simulink model "SOC_2023b"  (R2023b)
settings: Solver=VariableStepAuto  StartTime=0.0  StopTime=10.0  RelTol=1e-3
undefined symbols (not defined in the file or in its folder): Initial_SOC
(part 4 of 187)

== system SOC_2023b/SOC/Multi-Temperature SOC EKF51 ==
  note: McMaster Automotive Research Centre - MARC
      | Electrical Engineer: Romulo Navega Vieira, M.Sc and MBA - PhD Candidate
  Current : Inport
  Voltage : Inport  Port=2
  Temperature : Inport  Port=3
  Data Store Write1 : DataStoreWrite  DataStoreName=HVBat_SOCcell051  IndexOptions=Index vector (dialog)  Indices=1  OutputSizes=1
  Extended Kalman Filter - Multi Temperature EKF : Subsystem
  Gain : Gain  Gain=100
  Saturation : Saturate  UpperLimit=100  LowerLimit=0
  HVBat_SOCcell051 : Outport
  wires:
  Voltage/1 -> Extended Kalman Filter - Multi Temperature EKF/2(Voltage)
  Current/1 -> Extended Kalman Filter - Multi Temperature EKF/1(Current)
  Temperature/1 -> Extended Kalman Filter - Multi Temperature EKF/3(Temperature)
  Extended Kalman Filter - Multi Temperature EKF/1(SOC) -> Gain/1
  Gain/1 -> Saturation/1
  Saturation/1 -> HVBat_SOCcell051/1, Data Store Write1/1

== system SOC_2023b/SOC/Multi-Temperature SOC EKF52 ==
  note: McMaster Automotive Research Centre - MARC
      | Electrical Engineer: Romulo Navega Vieira, M.Sc and MBA - PhD Candidate
  Current : Inport
  Voltage : Inport  Port=2
  Temperature : Inport  Port=3
  Data Store Write1 : DataStoreWrite  DataStoreName=HVBat_SOCcell052  IndexOptions=Index vector (dialog)  Indices=1  OutputSizes=1
  Extended Kalman Filter - Multi Temperature EKF : Subsystem
  Gain : Gain  Gain=100
  Saturation : Saturate  UpperLimit=100  LowerLimit=0
  HVBat_SOCcell052 : Outport
  wires:
  Voltage/1 -> Extended Kalman Filter - Multi Temperature EKF/2(Voltage)
  Current/1 -> Extended Kalman Filter - Multi Temperature EKF/1(Current)
  Temperature/1 -> Extended Kalman Filter - Multi Temperature EKF/3(Temperature)
  Extended Kalman Filter - Multi Temperature EKF/1(SOC) -> Gain/1
  Gain/1 -> Saturation/1
  Saturation/1 -> HVBat_SOCcell052/1, Data Store Write1/1

== system SOC_2023b/SOC/Multi-Temperature SOC EKF53 ==
  note: McMaster Automotive Research Centre - MARC
      | Electrical Engineer: Romulo Navega Vieira, M.Sc and MBA - PhD Candidate
  Current : Inport
  Voltage : Inport  Port=2
  Temperature : Inport  Port=3
  Data Store Write1 : DataStoreWrite  DataStoreName=HVBat_SOCcell053  IndexOptions=Index vector (dialog)  Indices=1  OutputSizes=1
  Extended Kalman Filter - Multi Temperature EKF : Subsystem
  Gain : Gain  Gain=100
  Saturation : Saturate  UpperLimit=100  LowerLimit=0
  HVBat_SOCcell053 : Outport
  wires:
  Voltage/1 -> Extended Kalman Filter - Multi Temperature EKF/2(Voltage)
  Current/1 -> Extended Kalman Filter - Multi Temperature EKF/1(Current)
  Temperature/1 -> Extended Kalman Filter - Multi Temperature EKF/3(Temperature)
  Extended Kalman Filter - Multi Temperature EKF/1(SOC) -> Gain/1
  Gain/1 -> Saturation/1
  Saturation/1 -> HVBat_SOCcell053/1, Data Store Write1/1

== system SOC_2023b/SOC/Multi-Temperature SOC EKF54 ==
  note: McMaster Automotive Research Centre - MARC
      | Electrical Engineer: Romulo Navega Vieira, M.Sc and MBA - PhD Candidate
  Current : Inport
  Voltage : Inport  Port=2
  Temperature : Inport  Port=3
  Data Store Write1 : DataStoreWrite  DataStoreName=HVBat_SOCcell054  IndexOptions=Index vector (dialog)  Indices=1  OutputSizes=1
  Extended Kalman Filter - Multi Temperature EKF : Subsystem
  Gain : Gain  Gain=100
  Saturation : Saturate  UpperLimit=100  LowerLimit=0
  HVBat_SOCcell054 : Outport
  wires:
  Voltage/1 -> Extended Kalman Filter - Multi Temperature EKF/2(Voltage)
  Current/1 -> Extended Kalman Filter - Multi Temperature EKF/1(Current)
  Temperature/1 -> Extended Kalman Filter - Multi Temperature EKF/3(Temperature)
  Extended Kalman Filter - Multi Temperature EKF/1(SOC) -> Gain/1
  Gain/1 -> Saturation/1
  Saturation/1 -> HVBat_SOCcell054/1, Data Store Write1/1

== system SOC_2023b/SOC/Multi-Temperature SOC EKF55 ==
  note: McMaster Automotive Research Centre - MARC
      | Electrical Engineer: Romulo Navega Vieira, M.Sc and MBA - PhD Candidate
  Current : Inport
  Voltage : Inport  Port=2
  Temperature : Inport  Port=3
  Data Store Write1 : DataStoreWrite  DataStoreName=HVBat_SOCcell055  IndexOptions=Index vector (dialog)  Indices=1  OutputSizes=1
  Extended Kalman Filter - Multi Temperature EKF : Subsystem
  Gain : Gain  Gain=100
  Saturation : Saturate  UpperLimit=100  LowerLimit=0
  HVBat_SOCcell055 : Outport
  wires:
  Voltage/1 -> Extended Kalman Filter - Multi Temperature EKF/2(Voltage)
  Current/1 -> Extended Kalman Filter - Multi Temperature EKF/1(Current)
  Temperature/1 -> Extended Kalman Filter - Multi Temperature EKF/3(Temperature)
  Extended Kalman Filter - Multi Temperature EKF/1(SOC) -> Gain/1
  Gain/1 -> Saturation/1
  Saturation/1 -> HVBat_SOCcell055/1, Data Store Write1/1

== system SOC_2023b/SOC/Multi-Temperature SOC EKF56 ==
  note: McMaster Automotive Research Centre - MARC
      | Electrical Engineer: Romulo Navega Vieira, M.Sc and MBA - PhD Candidate
  Current : Inport
  Voltage : Inport  Port=2
  Temperature : Inport  Port=3
  Data Store Write1 : DataStoreWrite  DataStoreName=HVBat_SOCcell056  IndexOptions=Index vector (dialog)  Indices=1  OutputSizes=1
  Extended Kalman Filter - Multi Temperature EKF : Subsystem
  Gain : Gain  Gain=100
  Saturation : Saturate  UpperLimit=100  LowerLimit=0
  HVBat_SOCcell056 : Outport
  wires:
  Voltage/1 -> Extended Kalman Filter - Multi Temperature EKF/2(Voltage)
  Current/1 -> Extended Kalman Filter - Multi Temperature EKF/1(Current)
  Temperature/1 -> Extended Kalman Filter - Multi Temperature EKF/3(Temperature)
  Extended Kalman Filter - Multi Temperature EKF/1(SOC) -> Gain/1
  Gain/1 -> Saturation/1
  Saturation/1 -> HVBat_SOCcell056/1, Data Store Write1/1

== system SOC_2023b/SOC/Multi-Temperature SOC EKF57 ==
  note: McMaster Automotive Research Centre - MARC
      | Electrical Engineer: Romulo Navega Vieira, M.Sc and MBA - PhD Candidate
  Current : Inport
  Voltage : Inport  Port=2
  Temperature : Inport  Port=3
  Data Store Write1 : DataStoreWrite  DataStoreName=HVBat_SOCcell057  IndexOptions=Index vector (dialog)  Indices=1  OutputSizes=1
  Extended Kalman Filter - Multi Temperature EKF : Subsystem
  Gain : Gain  Gain=100
  Saturation : Saturate  UpperLimit=100  LowerLimit=0
  HVBat_SOCcell057 : Outport
  wires:
  Voltage/1 -> Extended Kalman Filter - Multi Temperature EKF/2(Voltage)
  Current/1 -> Extended Kalman Filter - Multi Temperature EKF/1(Current)
  Temperature/1 -> Extended Kalman Filter - Multi Temperature EKF/3(Temperature)
  Extended Kalman Filter - Multi Temperature EKF/1(SOC) -> Gain/1
  Gain/1 -> Saturation/1
  Saturation/1 -> HVBat_SOCcell057/1, Data Store Write1/1

== system SOC_2023b/SOC/Multi-Temperature SOC EKF58 ==
  note: McMaster Automotive Research Centre - MARC
      | Electrical Engineer: Romulo Navega Vieira, M.Sc and MBA - PhD Candidate
  Current : Inport
  Voltage : Inport  Port=2
  Temperature : Inport  Port=3
  Data Store Write1 : DataStoreWrite  DataStoreName=HVBat_SOCcell058  IndexOptions=Index vector (dialog)  Indices=1  OutputSizes=1
  Extended Kalman Filter - Multi Temperature EKF : Subsystem
  Gain : Gain  Gain=100
  Saturation : Saturate  UpperLimit=100  LowerLimit=0
  HVBat_SOCcell058 : Outport
  wires:
  Voltage/1 -> Extended Kalman Filter - Multi Temperature EKF/2(Voltage)
  Current/1 -> Extended Kalman Filter - Multi Temperature EKF/1(Current)
  Temperature/1 -> Extended Kalman Filter - Multi Temperature EKF/3(Temperature)
  Extended Kalman Filter - Multi Temperature EKF/1(SOC) -> Gain/1
  Gain/1 -> Saturation/1
  Saturation/1 -> HVBat_SOCcell058/1, Data Store Write1/1

== system SOC_2023b/SOC/Multi-Temperature SOC EKF59 ==
  note: McMaster Automotive Research Centre - MARC
      | Electrical Engineer: Romulo Navega Vieira, M.Sc and MBA - PhD Candidate
  Current : Inport
  Voltage : Inport  Port=2
  Temperature : Inport  Port=3
  Data Store Write1 : DataStoreWrite  DataStoreName=HVBat_SOCcell059  IndexOptions=Index vector (dialog)  Indices=1  OutputSizes=1
  Extended Kalman Filter - Multi Temperature EKF : Subsystem
  Gain : Gain  Gain=100
  Saturation : Saturate  UpperLimit=100  LowerLimit=0
  HVBat_SOCcell059 : Outport
  wires:
  Voltage/1 -> Extended Kalman Filter - Multi Temperature EKF/2(Voltage)
  Current/1 -> Extended Kalman Filter - Multi Temperature EKF/1(Current)
  Temperature/1 -> Extended Kalman Filter - Multi Temperature EKF/3(Temperature)
  Extended Kalman Filter - Multi Temperature EKF/1(SOC) -> Gain/1
  Gain/1 -> Saturation/1
  Saturation/1 -> HVBat_SOCcell059/1, Data Store Write1/1

== system SOC_2023b/SOC/Multi-Temperature SOC EKF6 ==
  note: McMaster Automotive Research Centre - MARC
      | Electrical Engineer: Romulo Navega Vieira, M.Sc and MBA - PhD Candidate
  Current : Inport
  Voltage : Inport  Port=2
  Temperature : Inport  Port=3
  Data Store Write1 : DataStoreWrite  DataStoreName=HVBat_SOCcell006  IndexOptions=Index vector (dialog)  Indices=1  OutputSizes=1
  Extended Kalman Filter - Multi Temperature EKF : Subsystem
  Gain : Gain  Gain=100
  Saturation : Saturate  UpperLimit=100  LowerLimit=0
  HVBat_SOCcell006 : Outport
  wires:
  Voltage/1 -> Extended Kalman Filter - Multi Temperature EKF/2(Voltage)
  Current/1 -> Extended Kalman Filter - Multi Temperature EKF/1(Current)
  Temperature/1 -> Extended Kalman Filter - Multi Temperature EKF/3(Temperature)
  Extended Kalman Filter - Multi Temperature EKF/1(SOC) -> Gain/1
  Gain/1 -> Saturation/1
  Saturation/1 -> HVBat_SOCcell006/1, Data Store Write1/1

== system SOC_2023b/SOC/Multi-Temperature SOC EKF60 ==
  note: McMaster Automotive Research Centre - MARC
      | Electrical Engineer: Romulo Navega Vieira, M.Sc and MBA - PhD Candidate
  Current : Inport
  Voltage : Inport  Port=2
  Temperature : Inport  Port=3
  Data Store Write1 : DataStoreWrite  DataStoreName=HVBat_SOCcell060  IndexOptions=Index vector (dialog)  Indices=1  OutputSizes=1
  Extended Kalman Filter - Multi Temperature EKF : Subsystem
  Gain : Gain  Gain=100
  Saturation : Saturate  UpperLimit=100  LowerLimit=0
  HVBat_SOCcell060 : Outport
  wires:
  Voltage/1 -> Extended Kalman Filter - Multi Temperature EKF/2(Voltage)
  Current/1 -> Extended Kalman Filter - Multi Temperature EKF/1(Current)
  Temperature/1 -> Extended Kalman Filter - Multi Temperature EKF/3(Temperature)
  Extended Kalman Filter - Multi Temperature EKF/1(SOC) -> Gain/1
  Gain/1 -> Saturation/1
  Saturation/1 -> HVBat_SOCcell060/1, Data Store Write1/1

== system SOC_2023b/SOC/Multi-Temperature SOC EKF61 ==
  note: McMaster Automotive Research Centre - MARC
      | Electrical Engineer: Romulo Navega Vieira, M.Sc and MBA - PhD Candidate
  Current : Inport
  Voltage : Inport  Port=2
  Temperature : Inport  Port=3
  Data Store Write1 : DataStoreWrite  DataStoreName=HVBat_SOCcell061  IndexOptions=Index vector (dialog)  Indices=1  OutputSizes=1
  Extended Kalman Filter - Multi Temperature EKF : Subsystem
  Gain : Gain  Gain=100
  Saturation : Saturate  UpperLimit=100  LowerLimit=0
  HVBat_SOCcell061 : Outport
  wires:
  Voltage/1 -> Extended Kalman Filter - Multi Temperature EKF/2(Voltage)
  Current/1 -> Extended Kalman Filter - Multi Temperature EKF/1(Current)
  Temperature/1 -> Extended Kalman Filter - Multi Temperature EKF/3(Temperature)
  Extended Kalman Filter - Multi Temperature EKF/1(SOC) -> Gain/1
  Gain/1 -> Saturation/1
  Saturation/1 -> HVBat_SOCcell061/1, Data Store Write1/1

== system SOC_2023b/SOC/Multi-Temperature SOC EKF62 ==
  note: McMaster Automotive Research Centre - MARC
      | Electrical Engineer: Romulo Navega Vieira, M.Sc and MBA - PhD Candidate
  Current : Inport
  Voltage : Inport  Port=2
  Temperature : Inport  Port=3
  Data Store Write1 : DataStoreWrite  DataStoreName=HVBat_SOCcell062  IndexOptions=Index vector (dialog)  Indices=1  OutputSizes=1
  Extended Kalman Filter - Multi Temperature EKF : Subsystem
  Gain : Gain  Gain=100
  Saturation : Saturate  UpperLimit=100  LowerLimit=0
  HVBat_SOCcell062 : Outport
  wires:
  Voltage/1 -> Extended Kalman Filter - Multi Temperature EKF/2(Voltage)
  Current/1 -> Extended Kalman Filter - Multi Temperature EKF/1(Current)
  Temperature/1 -> Extended Kalman Filter - Multi Temperature EKF/3(Temperature)
  Extended Kalman Filter - Multi Temperature EKF/1(SOC) -> Gain/1
  Gain/1 -> Saturation/1
  Saturation/1 -> HVBat_SOCcell062/1, Data Store Write1/1

== system SOC_2023b/SOC/Multi-Temperature SOC EKF63 ==
  note: McMaster Automotive Research Centre - MARC
      | Electrical Engineer: Romulo Navega Vieira, M.Sc and MBA - PhD Candidate
  Current : Inport
  Voltage : Inport  Port=2
  Temperature : Inport  Port=3
  Data Store Write1 : DataStoreWrite  DataStoreName=HVBat_SOCcell063  IndexOptions=Index vector (dialog)  Indices=1  OutputSizes=1
  Extended Kalman Filter - Multi Temperature EKF : Subsystem
  Gain : Gain  Gain=100
  Saturation : Saturate  UpperLimit=100  LowerLimit=0
  HVBat_SOCcell063 : Outport
  wires:
  Voltage/1 -> Extended Kalman Filter - Multi Temperature EKF/2(Voltage)
  Current/1 -> Extended Kalman Filter - Multi Temperature EKF/1(Current)
  Temperature/1 -> Extended Kalman Filter - Multi Temperature EKF/3(Temperature)
  Extended Kalman Filter - Multi Temperature EKF/1(SOC) -> Gain/1
  Gain/1 -> Saturation/1
  Saturation/1 -> HVBat_SOCcell063/1, Data Store Write1/1

== system SOC_2023b/SOC/Multi-Temperature SOC EKF64 ==
  note: McMaster Automotive Research Centre - MARC
      | Electrical Engineer: Romulo Navega Vieira, M.Sc and MBA - PhD Candidate
  Current : Inport
  Voltage : Inport  Port=2
  Temperature : Inport  Port=3
  Data Store Write1 : DataStoreWrite  DataStoreName=HVBat_SOCcell064  IndexOptions=Index vector (dialog)  Indices=1  OutputSizes=1
  Extended Kalman Filter - Multi Temperature EKF : Subsystem
  Gain : Gain  Gain=100
  Saturation : Saturate  UpperLimit=100  LowerLimit=0
  HVBat_SOCcell064 : Outport
  wires:
  Voltage/1 -> Extended Kalman Filter - Multi Temperature EKF/2(Voltage)
  Current/1 -> Extended Kalman Filter - Multi Temperature EKF/1(Current)
  Temperature/1 -> Extended Kalman Filter - Multi Temperature EKF/3(Temperature)
  Extended Kalman Filter - Multi Temperature EKF/1(SOC) -> Gain/1
  Gain/1 -> Saturation/1
  Saturation/1 -> HVBat_SOCcell064/1, Data Store Write1/1

== system SOC_2023b/SOC/Multi-Temperature SOC EKF65 ==
  note: McMaster Automotive Research Centre - MARC
      | Electrical Engineer: Romulo Navega Vieira, M.Sc and MBA - PhD Candidate
  Current : Inport
  Voltage : Inport  Port=2
  Temperature : Inport  Port=3
  Data Store Write1 : DataStoreWrite  DataStoreName=HVBat_SOCcell065  IndexOptions=Index vector (dialog)  Indices=1  OutputSizes=1
  Extended Kalman Filter - Multi Temperature EKF : Subsystem
  Gain : Gain  Gain=100
  Saturation : Saturate  UpperLimit=100  LowerLimit=0
  HVBat_SOCcell065 : Outport
  wires:
  Voltage/1 -> Extended Kalman Filter - Multi Temperature EKF/2(Voltage)
  Current/1 -> Extended Kalman Filter - Multi Temperature EKF/1(Current)
  Temperature/1 -> Extended Kalman Filter - Multi Temperature EKF/3(Temperature)
  Extended Kalman Filter - Multi Temperature EKF/1(SOC) -> Gain/1
  Gain/1 -> Saturation/1
  Saturation/1 -> HVBat_SOCcell065/1, Data Store Write1/1

== system SOC_2023b/SOC/Multi-Temperature SOC EKF66 ==
  note: McMaster Automotive Research Centre - MARC
      | Electrical Engineer: Romulo Navega Vieira, M.Sc and MBA - PhD Candidate
  Current : Inport
  Voltage : Inport  Port=2
  Temperature : Inport  Port=3
  Data Store Write1 : DataStoreWrite  DataStoreName=HVBat_SOCcell066  IndexOptions=Index vector (dialog)  Indices=1  OutputSizes=1
  Extended Kalman Filter - Multi Temperature EKF : Subsystem
  Gain : Gain  Gain=100
  Saturation : Saturate  UpperLimit=100  LowerLimit=0
  HVBat_SOCcell066 : Outport
  wires:
  Voltage/1 -> Extended Kalman Filter - Multi Temperature EKF/2(Voltage)
  Current/1 -> Extended Kalman Filter - Multi Temperature EKF/1(Current)
  Temperature/1 -> Extended Kalman Filter - Multi Temperature EKF/3(Temperature)
  Extended Kalman Filter - Multi Temperature EKF/1(SOC) -> Gain/1
  Gain/1 -> Saturation/1
  Saturation/1 -> HVBat_SOCcell066/1, Data Store Write1/1

== system SOC_2023b/SOC/Multi-Temperature SOC EKF67 ==
  note: McMaster Automotive Research Centre - MARC
      | Electrical Engineer: Romulo Navega Vieira, M.Sc and MBA - PhD Candidate
  Current : Inport
  Voltage : Inport  Port=2
  Temperature : Inport  Port=3
  Data Store Write1 : DataStoreWrite  DataStoreName=HVBat_SOCcell067  IndexOptions=Index vector (dialog)  Indices=1  OutputSizes=1
  Extended Kalman Filter - Multi Temperature EKF : Subsystem
  Gain : Gain  Gain=100
  Saturation : Saturate  UpperLimit=100  LowerLimit=0
  HVBat_SOCcell067 : Outport
  wires:
  Voltage/1 -> Extended Kalman Filter - Multi Temperature EKF/2(Voltage)
  Current/1 -> Extended Kalman Filter - Multi Temperature EKF/1(Current)
  Temperature/1 -> Extended Kalman Filter - Multi Temperature EKF/3(Temperature)
  Extended Kalman Filter - Multi Temperature EKF/1(SOC) -> Gain/1
  Gain/1 -> Saturation/1
  Saturation/1 -> HVBat_SOCcell067/1, Data Store Write1/1

== system SOC_2023b/SOC/Multi-Temperature SOC EKF68 ==
  note: McMaster Automotive Research Centre - MARC
      | Electrical Engineer: Romulo Navega Vieira, M.Sc and MBA - PhD Candidate
  Current : Inport
  Voltage : Inport  Port=2
  Temperature : Inport  Port=3
  Data Store Write1 : DataStoreWrite  DataStoreName=HVBat_SOCcell068  IndexOptions=Index vector (dialog)  Indices=1  OutputSizes=1
  Extended Kalman Filter - Multi Temperature EKF : Subsystem
  Gain : Gain  Gain=100
  Saturation : Saturate  UpperLimit=100  LowerLimit=0
  HVBat_SOCcell068 : Outport
  wires:
  Voltage/1 -> Extended Kalman Filter - Multi Temperature EKF/2(Voltage)
  Current/1 -> Extended Kalman Filter - Multi Temperature EKF/1(Current)
  Temperature/1 -> Extended Kalman Filter - Multi Temperature EKF/3(Temperature)
  Extended Kalman Filter - Multi Temperature EKF/1(SOC) -> Gain/1
  Gain/1 -> Saturation/1
  Saturation/1 -> HVBat_SOCcell068/1, Data Store Write1/1

== system SOC_2023b/SOC/Multi-Temperature SOC EKF69 ==
  note: McMaster Automotive Research Centre - MARC
      | Electrical Engineer: Romulo Navega Vieira, M.Sc and MBA - PhD Candidate
  Current : Inport
  Voltage : Inport  Port=2
  Temperature : Inport  Port=3
  Data Store Write1 : DataStoreWrite  DataStoreName=HVBat_SOCcell069  IndexOptions=Index vector (dialog)  Indices=1  OutputSizes=1
  Extended Kalman Filter - Multi Temperature EKF : Subsystem
  Gain : Gain  Gain=100
  Saturation : Saturate  UpperLimit=100  LowerLimit=0
  HVBat_SOCcell069 : Outport
  wires:
  Voltage/1 -> Extended Kalman Filter - Multi Temperature EKF/2(Voltage)
  Current/1 -> Extended Kalman Filter - Multi Temperature EKF/1(Current)
  Temperature/1 -> Extended Kalman Filter - Multi Temperature EKF/3(Temperature)
  Extended Kalman Filter - Multi Temperature EKF/1(SOC) -> Gain/1
  Gain/1 -> Saturation/1
  Saturation/1 -> HVBat_SOCcell069/1, Data Store Write1/1

== system SOC_2023b/SOC/Multi-Temperature SOC EKF7 ==
  note: McMaster Automotive Research Centre - MARC
      | Electrical Engineer: Romulo Navega Vieira, M.Sc and MBA - PhD Candidate
  Current : Inport
  Voltage : Inport  Port=2
  Temperature : Inport  Port=3
  Data Store Write1 : DataStoreWrite  DataStoreName=HVBat_SOCcell007  IndexOptions=Index vector (dialog)  Indices=1  OutputSizes=1
  Extended Kalman Filter - Multi Temperature EKF : Subsystem
  Gain : Gain  Gain=100
  Saturation : Saturate  UpperLimit=100  LowerLimit=0
  HVBat_SOCcell007 : Outport
  wires:
  Voltage/1 -> Extended Kalman Filter - Multi Temperature EKF/2(Voltage)
  Current/1 -> Extended Kalman Filter - Multi Temperature EKF/1(Current)
  Temperature/1 -> Extended Kalman Filter - Multi Temperature EKF/3(Temperature)
  Extended Kalman Filter - Multi Temperature EKF/1(SOC) -> Gain/1
  Gain/1 -> Saturation/1
  Saturation/1 -> HVBat_SOCcell007/1, Data Store Write1/1

== system SOC_2023b/SOC/Multi-Temperature SOC EKF70 ==
  note: McMaster Automotive Research Centre - MARC
      | Electrical Engineer: Romulo Navega Vieira, M.Sc and MBA - PhD Candidate
  Current : Inport
  Voltage : Inport  Port=2
  Temperature : Inport  Port=3
  Data Store Write1 : DataStoreWrite  DataStoreName=HVBat_SOCcell070  IndexOptions=Index vector (dialog)  Indices=1  OutputSizes=1
  Extended Kalman Filter - Multi Temperature EKF : Subsystem
  Gain : Gain  Gain=100
  Saturation : Saturate  UpperLimit=100  LowerLimit=0
  HVBat_SOCcell070 : Outport
  wires:
  Voltage/1 -> Extended Kalman Filter - Multi Temperature EKF/2(Voltage)
  Current/1 -> Extended Kalman Filter - Multi Temperature EKF/1(Current)
  Temperature/1 -> Extended Kalman Filter - Multi Temperature EKF/3(Temperature)
  Extended Kalman Filter - Multi Temperature EKF/1(SOC) -> Gain/1
  Gain/1 -> Saturation/1
  Saturation/1 -> HVBat_SOCcell070/1, Data Store Write1/1

== system SOC_2023b/SOC/Multi-Temperature SOC EKF71 ==
  note: McMaster Automotive Research Centre - MARC
      | Electrical Engineer: Romulo Navega Vieira, M.Sc and MBA - PhD Candidate
  Current : Inport
  Voltage : Inport  Port=2
  Temperature : Inport  Port=3
  Data Store Write1 : DataStoreWrite  DataStoreName=HVBat_SOCcell071  IndexOptions=Index vector (dialog)  Indices=1  OutputSizes=1
  Extended Kalman Filter - Multi Temperature EKF : Subsystem
  Gain : Gain  Gain=100
  Saturation : Saturate  UpperLimit=100  LowerLimit=0
  HVBat_SOCcell071 : Outport
  wires:
  Voltage/1 -> Extended Kalman Filter - Multi Temperature EKF/2(Voltage)
  Current/1 -> Extended Kalman Filter - Multi Temperature EKF/1(Current)
  Temperature/1 -> Extended Kalman Filter - Multi Temperature EKF/3(Temperature)
  Extended Kalman Filter - Multi Temperature EKF/1(SOC) -> Gain/1
  Gain/1 -> Saturation/1
  Saturation/1 -> HVBat_SOCcell071/1, Data Store Write1/1

== system SOC_2023b/SOC/Multi-Temperature SOC EKF72 ==
  note: McMaster Automotive Research Centre - MARC
      | Electrical Engineer: Romulo Navega Vieira, M.Sc and MBA - PhD Candidate
  Current : Inport
  Voltage : Inport  Port=2
  Temperature : Inport  Port=3
  Data Store Write1 : DataStoreWrite  DataStoreName=HVBat_SOCcell072  IndexOptions=Index vector (dialog)  Indices=1  OutputSizes=1
  Extended Kalman Filter - Multi Temperature EKF : Subsystem
  Gain : Gain  Gain=100
  Saturation : Saturate  UpperLimit=100  LowerLimit=0
  HVBat_SOCcell072 : Outport
  wires:
  Voltage/1 -> Extended Kalman Filter - Multi Temperature EKF/2(Voltage)
  Current/1 -> Extended Kalman Filter - Multi Temperature EKF/1(Current)
  Temperature/1 -> Extended Kalman Filter - Multi Temperature EKF/3(Temperature)
  Extended Kalman Filter - Multi Temperature EKF/1(SOC) -> Gain/1
  Gain/1 -> Saturation/1
  Saturation/1 -> HVBat_SOCcell072/1, Data Store Write1/1

== system SOC_2023b/SOC/Multi-Temperature SOC EKF73 ==
  note: McMaster Automotive Research Centre - MARC
      | Electrical Engineer: Romulo Navega Vieira, M.Sc and MBA - PhD Candidate
  Current : Inport
  Voltage : Inport  Port=2
  Temperature : Inport  Port=3
  Data Store Write1 : DataStoreWrite  DataStoreName=HVBat_SOCcell073  IndexOptions=Index vector (dialog)  Indices=1  OutputSizes=1
  Extended Kalman Filter - Multi Temperature EKF : Subsystem
  Gain : Gain  Gain=100
  Saturation : Saturate  UpperLimit=100  LowerLimit=0
  HVBat_SOCcell073 : Outport
  wires:
  Voltage/1 -> Extended Kalman Filter - Multi Temperature EKF/2(Voltage)
  Current/1 -> Extended Kalman Filter - Multi Temperature EKF/1(Current)
  Temperature/1 -> Extended Kalman Filter - Multi Temperature EKF/3(Temperature)
  Extended Kalman Filter - Multi Temperature EKF/1(SOC) -> Gain/1
  Gain/1 -> Saturation/1
  Saturation/1 -> HVBat_SOCcell073/1, Data Store Write1/1

== system SOC_2023b/SOC/Multi-Temperature SOC EKF74 ==
  note: McMaster Automotive Research Centre - MARC
      | Electrical Engineer: Romulo Navega Vieira, M.Sc and MBA - PhD Candidate
  Current : Inport
  Voltage : Inport  Port=2
  Temperature : Inport  Port=3
  Data Store Write1 : DataStoreWrite  DataStoreName=HVBat_SOCcell074  IndexOptions=Index vector (dialog)  Indices=1  OutputSizes=1
  Extended Kalman Filter - Multi Temperature EKF : Subsystem
  Gain : Gain  Gain=100
  Saturation : Saturate  UpperLimit=100  LowerLimit=0
  HVBat_SOCcell074 : Outport
  wires:
  Voltage/1 -> Extended Kalman Filter - Multi Temperature EKF/2(Voltage)
  Current/1 -> Extended Kalman Filter - Multi Temperature EKF/1(Current)
  Temperature/1 -> Extended Kalman Filter - Multi Temperature EKF/3(Temperature)
  Extended Kalman Filter - Multi Temperature EKF/1(SOC) -> Gain/1
  Gain/1 -> Saturation/1
  Saturation/1 -> HVBat_SOCcell074/1, Data Store Write1/1

== system SOC_2023b/SOC/Multi-Temperature SOC EKF75 ==
  note: McMaster Automotive Research Centre - MARC
      | Electrical Engineer: Romulo Navega Vieira, M.Sc and MBA - PhD Candidate
  Current : Inport
  Voltage : Inport  Port=2
  Temperature : Inport  Port=3
  Data Store Write1 : DataStoreWrite  DataStoreName=HVBat_SOCcell075  IndexOptions=Index vector (dialog)  Indices=1  OutputSizes=1
  Extended Kalman Filter - Multi Temperature EKF : Subsystem
  Gain : Gain  Gain=100
  Saturation : Saturate  UpperLimit=100  LowerLimit=0
  HVBat_SOCcell075 : Outport
  wires:
  Voltage/1 -> Extended Kalman Filter - Multi Temperature EKF/2(Voltage)
  Current/1 -> Extended Kalman Filter - Multi Temperature EKF/1(Current)
  Temperature/1 -> Extended Kalman Filter - Multi Temperature EKF/3(Temperature)
  Extended Kalman Filter - Multi Temperature EKF/1(SOC) -> Gain/1
  Gain/1 -> Saturation/1
  Saturation/1 -> HVBat_SOCcell075/1, Data Store Write1/1

== system SOC_2023b/SOC/Multi-Temperature SOC EKF76 ==
  note: McMaster Automotive Research Centre - MARC
      | Electrical Engineer: Romulo Navega Vieira, M.Sc and MBA - PhD Candidate
  Current : Inport
  Voltage : Inport  Port=2
  Temperature : Inport  Port=3
  Data Store Write1 : DataStoreWrite  DataStoreName=HVBat_SOCcell076  IndexOptions=Index vector (dialog)  Indices=1  OutputSizes=1
  Extended Kalman Filter - Multi Temperature EKF : Subsystem
  Gain : Gain  Gain=100
  Saturation : Saturate  UpperLimit=100  LowerLimit=0
  HVBat_SOCcell076 : Outport
  wires:
  Voltage/1 -> Extended Kalman Filter - Multi Temperature EKF/2(Voltage)
  Current/1 -> Extended Kalman Filter - Multi Temperature EKF/1(Current)
  Temperature/1 -> Extended Kalman Filter - Multi Temperature EKF/3(Temperature)
  Extended Kalman Filter - Multi Temperature EKF/1(SOC) -> Gain/1
  Gain/1 -> Saturation/1
  Saturation/1 -> HVBat_SOCcell076/1, Data Store Write1/1

== system SOC_2023b/SOC/Multi-Temperature SOC EKF77 ==
  note: McMaster Automotive Research Centre - MARC
      | Electrical Engineer: Romulo Navega Vieira, M.Sc and MBA - PhD Candidate
  Current : Inport
  Voltage : Inport  Port=2
  Temperature : Inport  Port=3
  Data Store Write1 : DataStoreWrite  DataStoreName=HVBat_SOCcell077  IndexOptions=Index vector (dialog)  Indices=1  OutputSizes=1
  Extended Kalman Filter - Multi Temperature EKF : Subsystem
  Gain : Gain  Gain=100
  Saturation : Saturate  UpperLimit=100  LowerLimit=0
  HVBat_SOCcell077 : Outport
  wires:
  Voltage/1 -> Extended Kalman Filter - Multi Temperature EKF/2(Voltage)
  Current/1 -> Extended Kalman Filter - Multi Temperature EKF/1(Current)
  Temperature/1 -> Extended Kalman Filter - Multi Temperature EKF/3(Temperature)
  Extended Kalman Filter - Multi Temperature EKF/1(SOC) -> Gain/1
  Gain/1 -> Saturation/1
  Saturation/1 -> HVBat_SOCcell077/1, Data Store Write1/1

== system SOC_2023b/SOC/Multi-Temperature SOC EKF78 ==
  note: McMaster Automotive Research Centre - MARC
      | Electrical Engineer: Romulo Navega Vieira, M.Sc and MBA - PhD Candidate
  Current : Inport
  Voltage : Inport  Port=2
  Temperature : Inport  Port=3
  Data Store Write1 : DataStoreWrite  DataStoreName=HVBat_SOCcell078  IndexOptions=Index vector (dialog)  Indices=1  OutputSizes=1
  Extended Kalman Filter - Multi Temperature EKF : Subsystem
  Gain : Gain  Gain=100
  Saturation : Saturate  UpperLimit=100  LowerLimit=0
  HVBat_SOCcell078 : Outport
  wires:
  Voltage/1 -> Extended Kalman Filter - Multi Temperature EKF/2(Voltage)
  Current/1 -> Extended Kalman Filter - Multi Temperature EKF/1(Current)
  Temperature/1 -> Extended Kalman Filter - Multi Temperature EKF/3(Temperature)
  Extended Kalman Filter - Multi Temperature EKF/1(SOC) -> Gain/1
  Gain/1 -> Saturation/1
  Saturation/1 -> HVBat_SOCcell078/1, Data Store Write1/1

== system SOC_2023b/SOC/Multi-Temperature SOC EKF79 ==
  note: McMaster Automotive Research Centre - MARC
      | Electrical Engineer: Romulo Navega Vieira, M.Sc and MBA - PhD Candidate
  Current : Inport
  Voltage : Inport  Port=2
  Temperature : Inport  Port=3
  Data Store Write1 : DataStoreWrite  DataStoreName=HVBat_SOCcell079  IndexOptions=Index vector (dialog)  Indices=1  OutputSizes=1
  Extended Kalman Filter - Multi Temperature EKF : Subsystem
  Gain : Gain  Gain=100
  Saturation : Saturate  UpperLimit=100  LowerLimit=0
  HVBat_SOCcell079 : Outport
  wires:
  Voltage/1 -> Extended Kalman Filter - Multi Temperature EKF/2(Voltage)
  Current/1 -> Extended Kalman Filter - Multi Temperature EKF/1(Current)
  Temperature/1 -> Extended Kalman Filter - Multi Temperature EKF/3(Temperature)
  Extended Kalman Filter - Multi Temperature EKF/1(SOC) -> Gain/1
  Gain/1 -> Saturation/1
  Saturation/1 -> HVBat_SOCcell079/1, Data Store Write1/1

== system SOC_2023b/SOC/Multi-Temperature SOC EKF8 ==
  note: McMaster Automotive Research Centre - MARC
      | Electrical Engineer: Romulo Navega Vieira, M.Sc and MBA - PhD Candidate
  Current : Inport
  Voltage : Inport  Port=2
  Temperature : Inport  Port=3
  Data Store Write1 : DataStoreWrite  DataStoreName=HVBat_SOCcell008  IndexOptions=Index vector (dialog)  Indices=1  OutputSizes=1
  Extended Kalman Filter - Multi Temperature EKF : Subsystem
  Gain : Gain  Gain=100
  Saturation : Saturate  UpperLimit=100  LowerLimit=0
  HVBat_SOCcell008 : Outport
  wires:
  Voltage/1 -> Extended Kalman Filter - Multi Temperature EKF/2(Voltage)
  Current/1 -> Extended Kalman Filter - Multi Temperature EKF/1(Current)
  Temperature/1 -> Extended Kalman Filter - Multi Temperature EKF/3(Temperature)
  Extended Kalman Filter - Multi Temperature EKF/1(SOC) -> Gain/1
  Gain/1 -> Saturation/1
  Saturation/1 -> HVBat_SOCcell008/1, Data Store Write1/1

== system SOC_2023b/SOC/Multi-Temperature SOC EKF80 ==
  note: McMaster Automotive Research Centre - MARC
      | Electrical Engineer: Romulo Navega Vieira, M.Sc and MBA - PhD Candidate
  Current : Inport
  Voltage : Inport  Port=2
  Temperature : Inport  Port=3
  Data Store Write1 : DataStoreWrite  DataStoreName=HVBat_SOCcell080  IndexOptions=Index vector (dialog)  Indices=1  OutputSizes=1
  Extended Kalman Filter - Multi Temperature EKF : Subsystem
  Gain : Gain  Gain=100
  Saturation : Saturate  UpperLimit=100  LowerLimit=0
  HVBat_SOCcell080 : Outport
  wires:
  Voltage/1 -> Extended Kalman Filter - Multi Temperature EKF/2(Voltage)
  Current/1 -> Extended Kalman Filter - Multi Temperature EKF/1(Current)
  Temperature/1 -> Extended Kalman Filter - Multi Temperature EKF/3(Temperature)
  Extended Kalman Filter - Multi Temperature EKF/1(SOC) -> Gain/1
  Gain/1 -> Saturation/1
  Saturation/1 -> HVBat_SOCcell080/1, Data Store Write1/1

== system SOC_2023b/SOC/Multi-Temperature SOC EKF81 ==
  note: McMaster Automotive Research Centre - MARC
      | Electrical Engineer: Romulo Navega Vieira, M.Sc and MBA - PhD Candidate
  Current : Inport
  Voltage : Inport  Port=2
  Temperature : Inport  Port=3
  Data Store Write1 : DataStoreWrite  DataStoreName=HVBat_SOCcell081  IndexOptions=Index vector (dialog)  Indices=1  OutputSizes=1
  Extended Kalman Filter - Multi Temperature EKF : Subsystem
  Gain : Gain  Gain=100
  Saturation : Saturate  UpperLimit=100  LowerLimit=0
  HVBat_SOCcell081 : Outport
  wires:
  Voltage/1 -> Extended Kalman Filter - Multi Temperature EKF/2(Voltage)
  Current/1 -> Extended Kalman Filter - Multi Temperature EKF/1(Current)
  Temperature/1 -> Extended Kalman Filter - Multi Temperature EKF/3(Temperature)
  Extended Kalman Filter - Multi Temperature EKF/1(SOC) -> Gain/1
  Gain/1 -> Saturation/1
  Saturation/1 -> HVBat_SOCcell081/1, Data Store Write1/1

== system SOC_2023b/SOC/Multi-Temperature SOC EKF82 ==
  note: McMaster Automotive Research Centre - MARC
      | Electrical Engineer: Romulo Navega Vieira, M.Sc and MBA - PhD Candidate
  Current : Inport
  Voltage : Inport  Port=2
  Temperature : Inport  Port=3
  Data Store Write1 : DataStoreWrite  DataStoreName=HVBat_SOCcell082  IndexOptions=Index vector (dialog)  Indices=1  OutputSizes=1
  Extended Kalman Filter - Multi Temperature EKF : Subsystem
  Gain : Gain  Gain=100
  Saturation : Saturate  UpperLimit=100  LowerLimit=0
  HVBat_SOCcell082 : Outport
  wires:
  Voltage/1 -> Extended Kalman Filter - Multi Temperature EKF/2(Voltage)
  Current/1 -> Extended Kalman Filter - Multi Temperature EKF/1(Current)
  Temperature/1 -> Extended Kalman Filter - Multi Temperature EKF/3(Temperature)
  Extended Kalman Filter - Multi Temperature EKF/1(SOC) -> Gain/1
  Gain/1 -> Saturation/1
  Saturation/1 -> HVBat_SOCcell082/1, Data Store Write1/1

== system SOC_2023b/SOC/Multi-Temperature SOC EKF83 ==
  note: McMaster Automotive Research Centre - MARC
      | Electrical Engineer: Romulo Navega Vieira, M.Sc and MBA - PhD Candidate
  Current : Inport
  Voltage : Inport  Port=2
  Temperature : Inport  Port=3
  Data Store Write1 : DataStoreWrite  DataStoreName=HVBat_SOCcell083  IndexOptions=Index vector (dialog)  Indices=1  OutputSizes=1
  Extended Kalman Filter - Multi Temperature EKF : Subsystem
  Gain : Gain  Gain=100
  Saturation : Saturate  UpperLimit=100  LowerLimit=0
  HVBat_SOCcell083 : Outport
  wires:
  Voltage/1 -> Extended Kalman Filter - Multi Temperature EKF/2(Voltage)
  Current/1 -> Extended Kalman Filter - Multi Temperature EKF/1(Current)
  Temperature/1 -> Extended Kalman Filter - Multi Temperature EKF/3(Temperature)
  Extended Kalman Filter - Multi Temperature EKF/1(SOC) -> Gain/1
  Gain/1 -> Saturation/1
  Saturation/1 -> HVBat_SOCcell083/1, Data Store Write1/1

== system SOC_2023b/SOC/Multi-Temperature SOC EKF84 ==
  note: McMaster Automotive Research Centre - MARC
      | Electrical Engineer: Romulo Navega Vieira, M.Sc and MBA - PhD Candidate
  Current : Inport
  Voltage : Inport  Port=2
  Temperature : Inport  Port=3
  Data Store Write1 : DataStoreWrite  DataStoreName=HVBat_SOCcell084  IndexOptions=Index vector (dialog)  Indices=1  OutputSizes=1
  Extended Kalman Filter - Multi Temperature EKF : Subsystem
  Gain : Gain  Gain=100
  Saturation : Saturate  UpperLimit=100  LowerLimit=0
  HVBat_SOCcell084 : Outport
  wires:
  Voltage/1 -> Extended Kalman Filter - Multi Temperature EKF/2(Voltage)
  Current/1 -> Extended Kalman Filter - Multi Temperature EKF/1(Current)
  Temperature/1 -> Extended Kalman Filter - Multi Temperature EKF/3(Temperature)
  Extended Kalman Filter - Multi Temperature EKF/1(SOC) -> Gain/1
  Gain/1 -> Saturation/1
  Saturation/1 -> HVBat_SOCcell084/1, Data Store Write1/1

== system SOC_2023b/SOC/Multi-Temperature SOC EKF85 ==
  note: McMaster Automotive Research Centre - MARC
      | Electrical Engineer: Romulo Navega Vieira, M.Sc and MBA - PhD Candidate
  Current : Inport
  Voltage : Inport  Port=2
  Temperature : Inport  Port=3
  Data Store Write1 : DataStoreWrite  DataStoreName=HVBat_SOCcell085  IndexOptions=Index vector (dialog)  Indices=1  OutputSizes=1
  Extended Kalman Filter - Multi Temperature EKF : Subsystem
  Gain : Gain  Gain=100
  Saturation : Saturate  UpperLimit=100  LowerLimit=0
  HVBat_SOCcell085 : Outport
  wires:
  Voltage/1 -> Extended Kalman Filter - Multi Temperature EKF/2(Voltage)
  Current/1 -> Extended Kalman Filter - Multi Temperature EKF/1(Current)
  Temperature/1 -> Extended Kalman Filter - Multi Temperature EKF/3(Temperature)
  Extended Kalman Filter - Multi Temperature EKF/1(SOC) -> Gain/1
  Gain/1 -> Saturation/1
  Saturation/1 -> HVBat_SOCcell085/1, Data Store Write1/1

== system SOC_2023b/SOC/Multi-Temperature SOC EKF86 ==
  note: McMaster Automotive Research Centre - MARC
      | Electrical Engineer: Romulo Navega Vieira, M.Sc and MBA - PhD Candidate
  Current : Inport
  Voltage : Inport  Port=2
  Temperature : Inport  Port=3
  Data Store Write1 : DataStoreWrite  DataStoreName=HVBat_SOCcell086  IndexOptions=Index vector (dialog)  Indices=1  OutputSizes=1
  Extended Kalman Filter - Multi Temperature EKF : Subsystem
  Gain : Gain  Gain=100
  Saturation : Saturate  UpperLimit=100  LowerLimit=0
  HVBat_SOCcell086 : Outport
  wires:
  Voltage/1 -> Extended Kalman Filter - Multi Temperature EKF/2(Voltage)
  Current/1 -> Extended Kalman Filter - Multi Temperature EKF/1(Current)
  Temperature/1 -> Extended Kalman Filter - Multi Temperature EKF/3(Temperature)
  Extended Kalman Filter - Multi Temperature EKF/1(SOC) -> Gain/1
  Gain/1 -> Saturation/1
  Saturation/1 -> HVBat_SOCcell086/1, Data Store Write1/1

== system SOC_2023b/SOC/Multi-Temperature SOC EKF87 ==
  note: McMaster Automotive Research Centre - MARC
      | Electrical Engineer: Romulo Navega Vieira, M.Sc and MBA - PhD Candidate
  Current : Inport
  Voltage : Inport  Port=2
  Temperature : Inport  Port=3
  Data Store Write1 : DataStoreWrite  DataStoreName=HVBat_SOCcell087  IndexOptions=Index vector (dialog)  Indices=1  OutputSizes=1
  Extended Kalman Filter - Multi Temperature EKF : Subsystem
  Gain : Gain  Gain=100
  Saturation : Saturate  UpperLimit=100  LowerLimit=0
  HVBat_SOCcell087 : Outport
  wires:
  Voltage/1 -> Extended Kalman Filter - Multi Temperature EKF/2(Voltage)
  Current/1 -> Extended Kalman Filter - Multi Temperature EKF/1(Current)
  Temperature/1 -> Extended Kalman Filter - Multi Temperature EKF/3(Temperature)
  Extended Kalman Filter - Multi Temperature EKF/1(SOC) -> Gain/1
  Gain/1 -> Saturation/1
  Saturation/1 -> HVBat_SOCcell087/1, Data Store Write1/1

== system SOC_2023b/SOC/Multi-Temperature SOC EKF88 ==
  note: McMaster Automotive Research Centre - MARC
      | Electrical Engineer: Romulo Navega Vieira, M.Sc and MBA - PhD Candidate
  Current : Inport
  Voltage : Inport  Port=2
  Temperature : Inport  Port=3
  Data Store Write1 : DataStoreWrite  DataStoreName=HVBat_SOCcell088  IndexOptions=Index vector (dialog)  Indices=1  OutputSizes=1
  Extended Kalman Filter - Multi Temperature EKF : Subsystem
  Gain : Gain  Gain=100
  Saturation : Saturate  UpperLimit=100  LowerLimit=0
  HVBat_SOCcell088 : Outport
  wires:
  Voltage/1 -> Extended Kalman Filter - Multi Temperature EKF/2(Voltage)
  Current/1 -> Extended Kalman Filter - Multi Temperature EKF/1(Current)
  Temperature/1 -> Extended Kalman Filter - Multi Temperature EKF/3(Temperature)
  Extended Kalman Filter - Multi Temperature EKF/1(SOC) -> Gain/1
  Gain/1 -> Saturation/1
  Saturation/1 -> HVBat_SOCcell088/1, Data Store Write1/1

== system SOC_2023b/SOC/Multi-Temperature SOC EKF89 ==
  note: McMaster Automotive Research Centre - MARC
      | Electrical Engineer: Romulo Navega Vieira, M.Sc and MBA - PhD Candidate
  Current : Inport
  Voltage : Inport  Port=2
  Temperature : Inport  Port=3
  Data Store Write1 : DataStoreWrite  DataStoreName=HVBat_SOCcell089  IndexOptions=Index vector (dialog)  Indices=1  OutputSizes=1
  Extended Kalman Filter - Multi Temperature EKF : Subsystem
  Gain : Gain  Gain=100
  Saturation : Saturate  UpperLimit=100  LowerLimit=0
  HVBat_SOCcell089 : Outport
  wires:
  Voltage/1 -> Extended Kalman Filter - Multi Temperature EKF/2(Voltage)
  Current/1 -> Extended Kalman Filter - Multi Temperature EKF/1(Current)
  Temperature/1 -> Extended Kalman Filter - Multi Temperature EKF/3(Temperature)
  Extended Kalman Filter - Multi Temperature EKF/1(SOC) -> Gain/1
  Gain/1 -> Saturation/1
  Saturation/1 -> HVBat_SOCcell089/1, Data Store Write1/1

== system SOC_2023b/SOC/Multi-Temperature SOC EKF9 ==
  note: McMaster Automotive Research Centre - MARC
      | Electrical Engineer: Romulo Navega Vieira, M.Sc and MBA - PhD Candidate
  Current : Inport
  Voltage : Inport  Port=2
  Temperature : Inport  Port=3
  Data Store Write1 : DataStoreWrite  DataStoreName=HVBat_SOCcell009  IndexOptions=Index vector (dialog)  Indices=1  OutputSizes=1
  Extended Kalman Filter - Multi Temperature EKF : Subsystem
  Gain : Gain  Gain=100
  Saturation : Saturate  UpperLimit=100  LowerLimit=0
  HVBat_SOCcell009 : Outport
  wires:
  Voltage/1 -> Extended Kalman Filter - Multi Temperature EKF/2(Voltage)
  Current/1 -> Extended Kalman Filter - Multi Temperature EKF/1(Current)
  Temperature/1 -> Extended Kalman Filter - Multi Temperature EKF/3(Temperature)
  Extended Kalman Filter - Multi Temperature EKF/1(SOC) -> Gain/1
  Gain/1 -> Saturation/1
  Saturation/1 -> HVBat_SOCcell009/1, Data Store Write1/1

== system SOC_2023b/SOC/Multi-Temperature SOC EKF90 ==
  note: McMaster Automotive Research Centre - MARC
      | Electrical Engineer: Romulo Navega Vieira, M.Sc and MBA - PhD Candidate
  Current : Inport
  Voltage : Inport  Port=2
  Temperature : Inport  Port=3
  Data Store Write1 : DataStoreWrite  DataStoreName=HVBat_SOCcell090  IndexOptions=Index vector (dialog)  Indices=1  OutputSizes=1
  Extended Kalman Filter - Multi Temperature EKF : Subsystem
  Gain : Gain  Gain=100
  Saturation : Saturate  UpperLimit=100  LowerLimit=0
  HVBat_SOCcell090 : Outport
  wires:
  Voltage/1 -> Extended Kalman Filter - Multi Temperature EKF/2(Voltage)
  Current/1 -> Extended Kalman Filter - Multi Temperature EKF/1(Current)
  Temperature/1 -> Extended Kalman Filter - Multi Temperature EKF/3(Temperature)
  Extended Kalman Filter - Multi Temperature EKF/1(SOC) -> Gain/1
  Gain/1 -> Saturation/1
  Saturation/1 -> HVBat_SOCcell090/1, Data Store Write1/1

== system SOC_2023b/SOC/Multi-Temperature SOC EKF91 ==
  note: McMaster Automotive Research Centre - MARC
      | Electrical Engineer: Romulo Navega Vieira, M.Sc and MBA - PhD Candidate
  Current : Inport
  Voltage : Inport  Port=2
  Temperature : Inport  Port=3
  Data Store Write1 : DataStoreWrite  DataStoreName=HVBat_SOCcell091  IndexOptions=Index vector (dialog)  Indices=1  OutputSizes=1
  Extended Kalman Filter - Multi Temperature EKF : Subsystem
  Gain : Gain  Gain=100
  Saturation : Saturate  UpperLimit=100  LowerLimit=0
  HVBat_SOCcell091 : Outport
  wires:
  Voltage/1 -> Extended Kalman Filter - Multi Temperature EKF/2(Voltage)
  Current/1 -> Extended Kalman Filter - Multi Temperature EKF/1(Current)
  Temperature/1 -> Extended Kalman Filter - Multi Temperature EKF/3(Temperature)
  Extended Kalman Filter - Multi Temperature EKF/1(SOC) -> Gain/1
  Gain/1 -> Saturation/1
  Saturation/1 -> HVBat_SOCcell091/1, Data Store Write1/1

== system SOC_2023b/SOC/Multi-Temperature SOC EKF92 ==
  note: McMaster Automotive Research Centre - MARC
      | Electrical Engineer: Romulo Navega Vieira, M.Sc and MBA - PhD Candidate
  Current : Inport
  Voltage : Inport  Port=2
  Temperature : Inport  Port=3
  Data Store Write1 : DataStoreWrite  DataStoreName=HVBat_SOCcell092  IndexOptions=Index vector (dialog)  Indices=1  OutputSizes=1
  Extended Kalman Filter - Multi Temperature EKF : Subsystem
  Gain : Gain  Gain=100
  Saturation : Saturate  UpperLimit=100  LowerLimit=0
  HVBat_SOCcell092 : Outport
  wires:
  Voltage/1 -> Extended Kalman Filter - Multi Temperature EKF/2(Voltage)
  Current/1 -> Extended Kalman Filter - Multi Temperature EKF/1(Current)
  Temperature/1 -> Extended Kalman Filter - Multi Temperature EKF/3(Temperature)
  Extended Kalman Filter - Multi Temperature EKF/1(SOC) -> Gain/1
  Gain/1 -> Saturation/1
  Saturation/1 -> HVBat_SOCcell092/1, Data Store Write1/1

== system SOC_2023b/SOC/Multi-Temperature SOC EKF93 ==
  note: McMaster Automotive Research Centre - MARC
      | Electrical Engineer: Romulo Navega Vieira, M.Sc and MBA - PhD Candidate
  Current : Inport
  Voltage : Inport  Port=2
  Temperature : Inport  Port=3
  Data Store Write1 : DataStoreWrite  DataStoreName=HVBat_SOCcell093  IndexOptions=Index vector (dialog)  Indices=1  OutputSizes=1
  Extended Kalman Filter - Multi Temperature EKF : Subsystem
  Gain : Gain  Gain=100
  Saturation : Saturate  UpperLimit=100  LowerLimit=0
  HVBat_SOCcell093 : Outport
  wires:
  Voltage/1 -> Extended Kalman Filter - Multi Temperature EKF/2(Voltage)
  Current/1 -> Extended Kalman Filter - Multi Temperature EKF/1(Current)
  Temperature/1 -> Extended Kalman Filter - Multi Temperature EKF/3(Temperature)
  Extended Kalman Filter - Multi Temperature EKF/1(SOC) -> Gain/1
  Gain/1 -> Saturation/1
  Saturation/1 -> HVBat_SOCcell093/1, Data Store Write1/1

== system SOC_2023b/SOC/Multi-Temperature SOC EKF94 ==
  note: McMaster Automotive Research Centre - MARC
      | Electrical Engineer: Romulo Navega Vieira, M.Sc and MBA - PhD Candidate
  Current : Inport
  Voltage : Inport  Port=2
  Temperature : Inport  Port=3
  Data Store Write1 : DataStoreWrite  DataStoreName=HVBat_SOCcell094  IndexOptions=Index vector (dialog)  Indices=1  OutputSizes=1
  Extended Kalman Filter - Multi Temperature EKF : Subsystem
  Gain : Gain  Gain=100
  Saturation : Saturate  UpperLimit=100  LowerLimit=0
  HVBat_SOCcell094 : Outport
  wires:
  Voltage/1 -> Extended Kalman Filter - Multi Temperature EKF/2(Voltage)
  Current/1 -> Extended Kalman Filter - Multi Temperature EKF/1(Current)
  Temperature/1 -> Extended Kalman Filter - Multi Temperature EKF/3(Temperature)
  Extended Kalman Filter - Multi Temperature EKF/1(SOC) -> Gain/1
  Gain/1 -> Saturation/1
  Saturation/1 -> HVBat_SOCcell094/1, Data Store Write1/1

== system SOC_2023b/SOC/Multi-Temperature SOC EKF95 ==
  note: McMaster Automotive Research Centre - MARC
      | Electrical Engineer: Romulo Navega Vieira, M.Sc and MBA - PhD Candidate
  Current : Inport
  Voltage : Inport  Port=2
  Temperature : Inport  Port=3
  Data Store Write1 : DataStoreWrite  DataStoreName=HVBat_SOCcell095  IndexOptions=Index vector (dialog)  Indices=1  OutputSizes=1
  Extended Kalman Filter - Multi Temperature EKF : Subsystem
  Gain : Gain  Gain=100
  Saturation : Saturate  UpperLimit=100  LowerLimit=0
  HVBat_SOCcell095 : Outport
  wires:
  Voltage/1 -> Extended Kalman Filter - Multi Temperature EKF/2(Voltage)
  Current/1 -> Extended Kalman Filter - Multi Temperature EKF/1(Current)
  Temperature/1 -> Extended Kalman Filter - Multi Temperature EKF/3(Temperature)
  Extended Kalman Filter - Multi Temperature EKF/1(SOC) -> Gain/1
  Gain/1 -> Saturation/1
  Saturation/1 -> HVBat_SOCcell095/1, Data Store Write1/1

== system SOC_2023b/SOC/Multi-Temperature SOC EKF96 ==
  note: McMaster Automotive Research Centre - MARC
      | Electrical Engineer: Romulo Navega Vieira, M.Sc and MBA - PhD Candidate
  Current : Inport
  Voltage : Inport  Port=2
  Temperature : Inport  Port=3
  Data Store Write1 : DataStoreWrite  DataStoreName=HVBat_SOCcell096  IndexOptions=Index vector (dialog)  Indices=1  OutputSizes=1
  Extended Kalman Filter - Multi Temperature EKF : Subsystem
  Gain : Gain  Gain=100
  Saturation : Saturate  UpperLimit=100  LowerLimit=0
  HVBat_SOCcell096 : Outport
  wires:
  Voltage/1 -> Extended Kalman Filter - Multi Temperature EKF/2(Voltage)
  Current/1 -> Extended Kalman Filter - Multi Temperature EKF/1(Current)
  Temperature/1 -> Extended Kalman Filter - Multi Temperature EKF/3(Temperature)
  Extended Kalman Filter - Multi Temperature EKF/1(SOC) -> Gain/1
  Gain/1 -> Saturation/1
  Saturation/1 -> HVBat_SOCcell096/1, Data Store Write1/1

== system SOC_2023b/SOC/Multi-Temperature SOC EKF97 ==
  note: McMaster Automotive Research Centre - MARC
      | Electrical Engineer: Romulo Navega Vieira, M.Sc and MBA - PhD Candidate
  Current : Inport
  Voltage : Inport  Port=2
  Temperature : Inport  Port=3
  Data Store Write1 : DataStoreWrite  DataStoreName=HVBat_SOCcell097  IndexOptions=Index vector (dialog)  Indices=1  OutputSizes=1
  Extended Kalman Filter - Multi Temperature EKF : Subsystem
  Gain : Gain  Gain=100
  Saturation : Saturate  UpperLimit=100  LowerLimit=0
  HVBat_SOCcell097 : Outport
  wires:
  Voltage/1 -> Extended Kalman Filter - Multi Temperature EKF/2(Voltage)
  Current/1 -> Extended Kalman Filter - Multi Temperature EKF/1(Current)
  Temperature/1 -> Extended Kalman Filter - Multi Temperature EKF/3(Temperature)
  Extended Kalman Filter - Multi Temperature EKF/1(SOC) -> Gain/1
  Gain/1 -> Saturation/1
  Saturation/1 -> HVBat_SOCcell097/1, Data Store Write1/1

== system SOC_2023b/SOC/Multi-Temperature SOC EKF98 ==
  note: McMaster Automotive Research Centre - MARC
      | Electrical Engineer: Romulo Navega Vieira, M.Sc and MBA - PhD Candidate
  Current : Inport
  Voltage : Inport  Port=2
  Temperature : Inport  Port=3
  Data Store Write1 : DataStoreWrite  DataStoreName=HVBat_SOCcell098  IndexOptions=Index vector (dialog)  Indices=1  OutputSizes=1
  Extended Kalman Filter - Multi Temperature EKF : Subsystem
  Gain : Gain  Gain=100
  Saturation : Saturate  UpperLimit=100  LowerLimit=0
  HVBat_SOCcell098 : Outport
  wires:
  Voltage/1 -> Extended Kalman Filter - Multi Temperature EKF/2(Voltage)
  Current/1 -> Extended Kalman Filter - Multi Temperature EKF/1(Current)
  Temperature/1 -> Extended Kalman Filter - Multi Temperature EKF/3(Temperature)
  Extended Kalman Filter - Multi Temperature EKF/1(SOC) -> Gain/1
  Gain/1 -> Saturation/1
  Saturation/1 -> HVBat_SOCcell098/1, Data Store Write1/1
